simulink model "l8_ver130"  (Simulink 7.9)
settings: Solver=FixedStepDiscrete  SolverMode=SingleTasking  StartTime=0.0  StopTime=10  RelTol=1e-3
undefined symbols (not defined in the file or in its folder): sfp
libraries: xbsIndex_r4 x20466, casper_library_misc x43, dspsigops x32, simulink x7, xps_library x5, casper_library_flow_control x4
(part 50 of 210)

== system l8_ver130/s2/Halfband3/adder_tree ==
  en_in : Inport
  din1 : Inport  Port=2
  din2 : Inport  Port=3
  din3 : Inport  Port=4
  din4 : Inport  Port=5
  din5 : Inport  Port=6
  din6 : Inport  Port=7
  din7 : Inport  Port=8
  din8 : Inport  Port=9
  din9 : Inport  Port=10
  addr1 : Subsystem
      settings: AttributesFormatString=|
      | hold period: 2
      |  add latency: 1
  addr11 : Subsystem
      settings: AttributesFormatString=|
      | hold period: 2
      |  add latency: 1
  addr2 : Subsystem
      settings: AttributesFormatString=|
      | hold period: 2
      |  add latency: 1
  addr3 : Subsystem
      settings: AttributesFormatString=|
      | hold period: 2
      |  add latency: 1
  addr4 : Subsystem
      settings: AttributesFormatString=|
      | hold period: 2
      |  add latency: 1
  addr6 : Subsystem
      settings: AttributesFormatString=|
      | hold period: 2
      |  add latency: 1
  addr7 : Subsystem
      settings: AttributesFormatString=|
      | hold period: 2
      |  add latency: 1
  addr9 : Subsystem
      settings: AttributesFormatString=|
      | hold period: 2
      |  add latency: 1
  dly10 : xbsIndex_r4/Delay  rst=off  infoeditControl=Selection of Reset will increase slice count due to use of real FFs and instead of SRLs  en=off  latency=2  dbl_ovrd=off  reg_retiming=on  block_type=delay
  dly5 : xbsIndex_r4/Delay  rst=off  infoeditControl=Selection of Reset will increase slice count due to use of real FFs and instead of SRLs  en=off  latency=2  dbl_ovrd=off  reg_retiming=on  block_type=delay
  dly8 : xbsIndex_r4/Delay  rst=off  infoeditControl=Selection of Reset will increase slice count due to use of real FFs and instead of SRLs  en=off  latency=2  dbl_ovrd=off  reg_retiming=on  block_type=delay
  sync_delay : xbsIndex_r4/Delay  rst=off  infoeditControl=Selection of Reset will increase slice count due to use of real FFs and instead of SRLs  en=off  latency=4  dbl_ovrd=off  reg_retiming=on  block_type=delay
  en_out : Outport
  dout : Outport  Port=2
  wires:
  en_in/1 -> addr11/3(en_in), addr9/3(en_in), addr7/3(en_in), addr6/3(en_in), addr4/3(en_in), addr3/3(en_in), addr2/3(en_in), addr1/3(en_in), sync_delay/1
  addr11/1(sum_ab) -> dout/1  "adder_outs4_1"
  dly10/1 -> addr11/2(data_b)  "adder_outs3_2"
  addr9/1(sum_ab) -> addr11/1(data_a)  "adder_outs3_1"
  dly8/1 -> dly10/1  "adder_outs2_3"
  addr7/1(sum_ab) -> addr9/2(data_b)  "adder_outs2_2"
  addr6/1(sum_ab) -> addr9/1(data_a)  "adder_outs2_1"
  dly5/1 -> dly8/1  "adder_outs1_5"
  addr4/1(sum_ab) -> addr7/2(data_b)  "adder_outs1_4"
  addr3/1(sum_ab) -> addr7/1(data_a)  "adder_outs1_3"
  addr2/1(sum_ab) -> addr6/2(data_b)  "adder_outs1_2"
  addr1/1(sum_ab) -> addr6/1(data_a)  "adder_outs1_1"
  din9/1 -> dly5/1
  din8/1 -> addr4/2(data_b)
  din7/1 -> addr4/1(data_a)
  din6/1 -> addr3/2(data_b)
  din5/1 -> addr3/1(data_a)
  din4/1 -> addr2/2(data_b)
  din3/1 -> addr2/1(data_a)
  din2/1 -> addr1/2(data_b)
  din1/1 -> addr1/1(data_a)
  sync_delay/1 -> en_out/1

== system l8_ver130/s2/Halfband3/en_gen ==
  sync_in : Inport
  Constant : xbsIndex_r4/Constant  const=0  gui_display_data_type=Fixed-point  arith_type=Signed (2's comp)  n_bits=16  bin_pt=0  preci_type=Single  exp_width=8  frac_width=24  explicit_period=on  period=1  equ=P=C  opselect=C  inp2=PCIN>>17  opr=+  inp1=P  carry=CIN  dbl_ovrd=off  block_type=constant
  Counter1 : xbsIndex_r4/Counter  cnt_type=Count Limited  cnt_to=0  operation=Down  start_count=1  cnt_by_val=1  arith_type=Unsigned  n_bits=1  bin_pt=0  load_pin=off  rst=on  en=off  explicit_period=on  period=1  dbl_ovrd=off  use_behavioral_HDL=off  implementation=Fabric  block_type=counter
  Counter_delay : xbsIndex_r4/Delay  rst=off  infoeditControl=Selection of Reset will increase slice count due to use of real FFs and instead of SRLs  en=off  latency=0  dbl_ovrd=off  reg_retiming=off  block_type=delay
  Logical : xbsIndex_r4/Logical  logical_function=OR  inputs=2  en=off  latency=1  precision=Full  arith_type=Unsigned  n_bits=16  bin_pt=0  align_bp=on  dbl_ovrd=off  block_type=logical
  Relational : xbsIndex_r4/Relational  mode=a=b  en=off  latency=1  dbl_ovrd=off  block_type=relational
  en_out : Outport
  wires:
  Logical/1 -> en_out/1, Counter1/1  "xlsub1_Logical_out1"
  Relational/1 -> Logical/1  "xlsub1_Relational_out1"
  Constant/1 -> Relational/2  "xlsub1_Constant_out1"
  Counter_delay/1 -> Relational/1  "counter_delay_out"
  sync_in/1 -> Logical/2
  Counter1/1 -> Counter_delay/1  "xlsub1_Counter1_out1"

== system l8_ver130/s2/Halfband3/hold_fir_tap1 ==
  data_in : Inport
  en_in : Inport  Port=2
  Mult : xbsIndex_r4/Mult  precision=Full  arith_type="Signed  (2's comp)"  n_bits=16  bin_pt=14  quantization=Truncate  overflow=Wrap  en=on  latency=3  dbl_ovrd=off  use_behavioral_HDL=off  opt=Speed  use_embedded=on  optimum_pipeline=off  pipeline=on  use_rpm=on  placement_style=Triangular  block_type=mult
  coefficient : xbsIndex_r4/Constant  const=-0.026472867764434  gui_display_data_type=Fixed-point  arith_type=Signed (2's comp)  n_bits=16  bin_pt=14  preci_type=Single  exp_width=8  frac_width=24  explicit_period=on  period=1  equ=P=C  opselect=C  inp2=PCIN>>17  opr=+  inp1=P  carry=CIN  dbl_ovrd=off  block_type=constant
  mult_out : Outport
  en_out : Outport  Port=2
  wires:
  en_in/1 -> en_out/1, Mult/3
  coefficient/1 -> Mult/1  "xlsub2_coefficient_out1"
  Mult/1 -> mult_out/1
  data_in/1 -> Mult/2

== system l8_ver130/s2/Halfband3/hold_fir_tap11 ==
  data_in : Inport
  en_in : Inport  Port=2
  Mult : xbsIndex_r4/Mult  precision=Full  arith_type="Signed  (2's comp)"  n_bits=16  bin_pt=14  quantization=Truncate  overflow=Wrap  en=on  latency=3  dbl_ovrd=off  use_behavioral_HDL=off  opt=Speed  use_embedded=on  optimum_pipeline=off  pipeline=on  use_rpm=on  placement_style=Triangular  block_type=mult
  coefficient : xbsIndex_r4/Constant  const=-0.0934071809736732  gui_display_data_type=Fixed-point  arith_type=Signed (2's comp)  n_bits=16  bin_pt=14  preci_type=Single  exp_width=8  frac_width=24  explicit_period=on  period=1  equ=P=C  opselect=C  inp2=PCIN>>17  opr=+  inp1=P  carry=CIN  dbl_ovrd=off  block_type=constant
  mult_out : Outport
  en_out : Outport  Port=2
  wires:
  en_in/1 -> en_out/1, Mult/3
  coefficient/1 -> Mult/1  "xlsub2_coefficient_out1"
  Mult/1 -> mult_out/1
  data_in/1 -> Mult/2

== system l8_ver130/s2/Halfband3/hold_fir_tap13 ==
  data_in : Inport
  en_in : Inport  Port=2
  Mult : xbsIndex_r4/Mult  precision=Full  arith_type="Signed  (2's comp)"  n_bits=16  bin_pt=14  quantization=Truncate  overflow=Wrap  en=on  latency=3  dbl_ovrd=off  use_behavioral_HDL=off  opt=Speed  use_embedded=on  optimum_pipeline=off  pipeline=on  use_rpm=on  placement_style=Triangular  block_type=mult
  coefficient : xbsIndex_r4/Constant  const=0.0440903441068704  gui_display_data_type=Fixed-point  arith_type=Signed (2's comp)  n_bits=16  bin_pt=14  preci_type=Single  exp_width=8  frac_width=24  explicit_period=on  period=1  equ=P=C  opselect=C  inp2=PCIN>>17  opr=+  inp1=P  carry=CIN  dbl_ovrd=off  block_type=constant
  mult_out : Outport
  en_out : Outport  Port=2
  wires:
  en_in/1 -> en_out/1, Mult/3
  coefficient/1 -> Mult/1  "xlsub2_coefficient_out1"
  Mult/1 -> mult_out/1
  data_in/1 -> Mult/2

== system l8_ver130/s2/Halfband3/hold_fir_tap15 ==
  data_in : Inport
  en_in : Inport  Port=2
  Mult : xbsIndex_r4/Mult  precision=Full  arith_type="Signed  (2's comp)"  n_bits=16  bin_pt=14  quantization=Truncate  overflow=Wrap  en=on  latency=3  dbl_ovrd=off  use_behavioral_HDL=off  opt=Speed  use_embedded=on  optimum_pipeline=off  pipeline=on  use_rpm=on  placement_style=Triangular  block_type=mult
  coefficient : xbsIndex_r4/Constant  const=-0.026472867764434  gui_display_data_type=Fixed-point  arith_type=Signed (2's comp)  n_bits=16  bin_pt=14  preci_type=Single  exp_width=8  frac_width=24  explicit_period=on  period=1  equ=P=C  opselect=C  inp2=PCIN>>17  opr=+  inp1=P  carry=CIN  dbl_ovrd=off  block_type=constant
  mult_out : Outport
  en_out : Outport  Port=2
  wires:
  en_in/1 -> en_out/1, Mult/3
  coefficient/1 -> Mult/1  "xlsub2_coefficient_out1"
  Mult/1 -> mult_out/1
  data_in/1 -> Mult/2

== system l8_ver130/s2/Halfband3/hold_fir_tap3 ==
  data_in : Inport
  en_in : Inport  Port=2
  Mult : xbsIndex_r4/Mult  precision=Full  arith_type="Signed  (2's comp)"  n_bits=16  bin_pt=14  quantization=Truncate  overflow=Wrap  en=on  latency=3  dbl_ovrd=off  use_behavioral_HDL=off  opt=Speed  use_embedded=on  optimum_pipeline=off  pipeline=on  use_rpm=on  placement_style=Triangular  block_type=mult
  coefficient : xbsIndex_r4/Constant  const=0.0440903441068704  gui_display_data_type=Fixed-point  arith_type=Signed (2's comp)  n_bits=16  bin_pt=14  preci_type=Single  exp_width=8  frac_width=24  explicit_period=on  period=1  equ=P=C  opselect=C  inp2=PCIN>>17  opr=+  inp1=P  carry=CIN  dbl_ovrd=off  block_type=constant
  mult_out : Outport
  en_out : Outport  Port=2
  wires:
  en_in/1 -> en_out/1, Mult/3
  coefficient/1 -> Mult/1  "xlsub2_coefficient_out1"
  Mult/1 -> mult_out/1
  data_in/1 -> Mult/2

== system l8_ver130/s2/Halfband3/hold_fir_tap5 ==
  data_in : Inport
  en_in : Inport  Port=2
  Mult : xbsIndex_r4/Mult  precision=Full  arith_type="Signed  (2's comp)"  n_bits=16  bin_pt=14  quantization=Truncate  overflow=Wrap  en=on  latency=3  dbl_ovrd=off  use_behavioral_HDL=off  opt=Speed  use_embedded=on  optimum_pipeline=off  pipeline=on  use_rpm=on  placement_style=Triangular  block_type=mult
  coefficient : xbsIndex_r4/Constant  const=-0.0934071809736732  gui_display_data_type=Fixed-point  arith_type=Signed (2's comp)  n_bits=16  bin_pt=14  preci_type=Single  exp_width=8  frac_width=24  explicit_period=on  period=1  equ=P=C  opselect=C  inp2=PCIN>>17  opr=+  inp1=P  carry=CIN  dbl_ovrd=off  block_type=constant
  mult_out : Outport
  en_out : Outport  Port=2
  wires:
  en_in/1 -> en_out/1, Mult/3
  coefficient/1 -> Mult/1  "xlsub2_coefficient_out1"
  Mult/1 -> mult_out/1
  data_in/1 -> Mult/2

== system l8_ver130/s2/Halfband3/hold_fir_tap7 ==
  data_in : Inport
  en_in : Inport  Port=2
  Mult : xbsIndex_r4/Mult  precision=Full  arith_type="Signed  (2's comp)"  n_bits=16  bin_pt=14  quantization=Truncate  overflow=Wrap  en=on  latency=3  dbl_ovrd=off  use_behavioral_HDL=off  opt=Speed  use_embedded=on  optimum_pipeline=off  pipeline=on  use_rpm=on  placement_style=Triangular  block_type=mult
  coefficient : xbsIndex_r4/Constant  const=0.313910410160698  gui_display_data_type=Fixed-point  arith_type=Signed (2's comp)  n_bits=16  bin_pt=14  preci_type=Single  exp_width=8  frac_width=24  explicit_period=on  period=1  equ=P=C  opselect=C  inp2=PCIN>>17  opr=+  inp1=P  carry=CIN  dbl_ovrd=off  block_type=constant
  mult_out : Outport
  en_out : Outport  Port=2
  wires:
  en_in/1 -> en_out/1, Mult/3
  coefficient/1 -> Mult/1  "xlsub2_coefficient_out1"
  Mult/1 -> mult_out/1
  data_in/1 -> Mult/2

== system l8_ver130/s2/Halfband3/hold_fir_tap8 ==
  data_in : Inport
  en_in : Inport  Port=2
  Mult : xbsIndex_r4/Mult  precision=Full  arith_type="Signed  (2's comp)"  n_bits=16  bin_pt=14  quantization=Truncate  overflow=Wrap  en=on  latency=3  dbl_ovrd=off  use_behavioral_HDL=off  opt=Speed  use_embedded=on  optimum_pipeline=off  pipeline=on  use_rpm=on  placement_style=Triangular  block_type=mult
  coefficient : xbsIndex_r4/Constant  const=0.5  gui_display_data_type=Fixed-point  arith_type=Signed (2's comp)  n_bits=16  bin_pt=14  preci_type=Single  exp_width=8  frac_width=24  explicit_period=on  period=1  equ=P=C  opselect=C  inp2=PCIN>>17  opr=+  inp1=P  carry=CIN  dbl_ovrd=off  block_type=constant
  mult_out : Outport
  en_out : Outport  Port=2
  wires:
  en_in/1 -> en_out/1, Mult/3
  coefficient/1 -> Mult/1  "xlsub2_coefficient_out1"
  Mult/1 -> mult_out/1
  data_in/1 -> Mult/2

== system l8_ver130/s2/Halfband3/hold_fir_tap9 ==
  data_in : Inport
  en_in : Inport  Port=2
  Mult : xbsIndex_r4/Mult  precision=Full  arith_type="Signed  (2's comp)"  n_bits=16  bin_pt=14  quantization=Truncate  overflow=Wrap  en=on  latency=3  dbl_ovrd=off  use_behavioral_HDL=off  opt=Speed  use_embedded=on  optimum_pipeline=off  pipeline=on  use_rpm=on  placement_style=Triangular  block_type=mult
  coefficient : xbsIndex_r4/Constant  const=0.313910410160698  gui_display_data_type=Fixed-point  arith_type=Signed (2's comp)  n_bits=16  bin_pt=14  preci_type=Single  exp_width=8  frac_width=24  explicit_period=on  period=1  equ=P=C  opselect=C  inp2=PCIN>>17  opr=+  inp1=P  carry=CIN  dbl_ovrd=off  block_type=constant
  mult_out : Outport
  en_out : Outport  Port=2
  wires:
  en_in/1 -> en_out/1, Mult/3
  coefficient/1 -> Mult/1  "xlsub2_coefficient_out1"
  Mult/1 -> mult_out/1
  data_in/1 -> Mult/2

== system l8_ver130/s2/cic_pow2_0/Stage1_dec2 ==
  in1 : Inport
  in2 : Inport  Port=2
  in3 : Inport  Port=3
  in4 : Inport  Port=4
  in5 : Inport  Port=5
  in6 : Inport  Port=6
  in7 : Inport  Port=7
  in8 : Inport  Port=8
  in9 : Inport  Port=9
  in10 : Inport  Port=10
  in11 : Inport  Port=11
  in12 : Inport  Port=12
  in13 : Inport  Port=13
  in14 : Inport  Port=14
  in15 : Inport  Port=15
  in16 : Inport  Port=16
  sync_in : Inport  Port=17
  p_adder1 : Subsystem
      settings: AttributesFormatString=|
      | Accumulation Length = 2
      | Add Latency = 1
      | Delay Length =1
  p_adder2 : Subsystem
      settings: AttributesFormatString=|
      | Accumulation Length = 2
      | Add Latency = 1
      | Delay Length =1
  p_adder3 : Subsystem
      settings: AttributesFormatString=|
      | Accumulation Length = 2
      | Add Latency = 1
      | Delay Length =1
  p_adder4 : Subsystem
      settings: AttributesFormatString=|
      | Accumulation Length = 2
      | Add Latency = 1
      | Delay Length =1
  out1 : Outport
  out2 : Outport  Port=2
  out3 : Outport  Port=3
  out4 : Outport  Port=4
  out5 : Outport  Port=5
  out6 : Outport  Port=6
  out7 : Outport  Port=7
  out8 : Outport  Port=8
  sync_out : Outport  Port=9
  wires:
  sync_in/1 -> p_adder4/1(sync_in), p_adder3/1(sync_in), p_adder2/1(sync_in), p_adder1/1(sync_in)
  p_adder4/1(sync_out) -> sync_out/1  "sync_in5"
  p_adder4/17(out16) -> out8/1  "pa_i5_16"
  p_adder4/15(out14) -> out7/1  "pa_i5_14"
  p_adder4/13(out12) -> out6/1  "pa_i5_12"
  p_adder4/11(out10) -> out5/1  "pa_i5_10"
  p_adder4/9(out8) -> out4/1  "pa_i5_8"
  p_adder4/7(out6) -> out3/1  "pa_i5_6"
  p_adder4/5(out4) -> out2/1  "pa_i5_4"
  p_adder4/3(out2) -> out1/1  "pa_i5_2"
  p_adder3/17(out16) -> p_adder4/17(in16)  "pa_i4_16"
  p_adder3/16(out15) -> p_adder4/16(in15)  "pa_i4_15"
  p_adder3/15(out14) -> p_adder4/15(in14)  "pa_i4_14"
  p_adder3/14(out13) -> p_adder4/14(in13)  "pa_i4_13"
  p_adder3/13(out12) -> p_adder4/13(in12)  "pa_i4_12"
  p_adder3/12(out11) -> p_adder4/12(in11)  "pa_i4_11"
  p_adder3/11(out10) -> p_adder4/11(in10)  "pa_i4_10"
  p_adder3/10(out9) -> p_adder4/10(in9)  "pa_i4_9"
  p_adder3/9(out8) -> p_adder4/9(in8)  "pa_i4_8"
  p_adder3/8(out7) -> p_adder4/8(in7)  "pa_i4_7"
  p_adder3/7(out6) -> p_adder4/7(in6)  "pa_i4_6"
  p_adder3/6(out5) -> p_adder4/6(in5)  "pa_i4_5"
  p_adder3/5(out4) -> p_adder4/5(in4)  "pa_i4_4"
  p_adder3/4(out3) -> p_adder4/4(in3)  "pa_i4_3"
  p_adder3/3(out2) -> p_adder4/3(in2)  "pa_i4_2"
  p_adder3/2(out1) -> p_adder4/2(in1)  "pa_i4_1"
  p_adder2/17(out16) -> p_adder3/17(in16)  "pa_i3_16"
  p_adder2/16(out15) -> p_adder3/16(in15)  "pa_i3_15"
  p_adder2/15(out14) -> p_adder3/15(in14)  "pa_i3_14"
  p_adder2/14(out13) -> p_adder3/14(in13)  "pa_i3_13"
  p_adder2/13(out12) -> p_adder3/13(in12)  "pa_i3_12"
  p_adder2/12(out11) -> p_adder3/12(in11)  "pa_i3_11"
  p_adder2/11(out10) -> p_adder3/11(in10)  "pa_i3_10"
  p_adder2/10(out9) -> p_adder3/10(in9)  "pa_i3_9"
  p_adder2/9(out8) -> p_adder3/9(in8)  "pa_i3_8"
  p_adder2/8(out7) -> p_adder3/8(in7)  "pa_i3_7"
  p_adder2/7(out6) -> p_adder3/7(in6)  "pa_i3_6"
  p_adder2/6(out5) -> p_adder3/6(in5)  "pa_i3_5"
  p_adder2/5(out4) -> p_adder3/5(in4)  "pa_i3_4"
  p_adder2/4(out3) -> p_adder3/4(in3)  "pa_i3_3"
  p_adder2/3(out2) -> p_adder3/3(in2)  "pa_i3_2"
  p_adder2/2(out1) -> p_adder3/2(in1)  "pa_i3_1"
  p_adder1/17(out16) -> p_adder2/17(in16)  "pa_i2_16"
  p_adder1/16(out15) -> p_adder2/16(in15)  "pa_i2_15"
  p_adder1/15(out14) -> p_adder2/15(in14)  "pa_i2_14"
  p_adder1/14(out13) -> p_adder2/14(in13)  "pa_i2_13"
  p_adder1/13(out12) -> p_adder2/13(in12)  "pa_i2_12"
  p_adder1/12(out11) -> p_adder2/12(in11)  "pa_i2_11"
  p_adder1/11(out10) -> p_adder2/11(in10)  "pa_i2_10"
  p_adder1/10(out9) -> p_adder2/10(in9)  "pa_i2_9"
  p_adder1/9(out8) -> p_adder2/9(in8)  "pa_i2_8"
  p_adder1/8(out7) -> p_adder2/8(in7)  "pa_i2_7"
  p_adder1/7(out6) -> p_adder2/7(in6)  "pa_i2_6"
  p_adder1/6(out5) -> p_adder2/6(in5)  "pa_i2_5"
  p_adder1/5(out4) -> p_adder2/5(in4)  "pa_i2_4"
  p_adder1/4(out3) -> p_adder2/4(in3)  "pa_i2_3"
  p_adder1/3(out2) -> p_adder2/3(in2)  "pa_i2_2"
  p_adder1/2(out1) -> p_adder2/2(in1)  "pa_i2_1"
  in16/1 -> p_adder1/17(in16)
  in15/1 -> p_adder1/16(in15)
  in14/1 -> p_adder1/15(in14)
  in13/1 -> p_adder1/14(in13)
  in12/1 -> p_adder1/13(in12)
  in11/1 -> p_adder1/12(in11)
  in10/1 -> p_adder1/11(in10)
  in9/1 -> p_adder1/10(in9)
  in8/1 -> p_adder1/9(in8)
  in7/1 -> p_adder1/8(in7)
  in6/1 -> p_adder1/7(in6)
  in5/1 -> p_adder1/6(in5)
  in4/1 -> p_adder1/5(in4)
  in3/1 -> p_adder1/4(in3)
  in2/1 -> p_adder1/3(in2)
  in1/1 -> p_adder1/2(in1)

== system l8_ver130/s2/cic_pow2_0/Stage2_dec2 ==
  in1 : Inport
  in2 : Inport  Port=2
  in3 : Inport  Port=3
  in4 : Inport  Port=4
  in5 : Inport  Port=5
  in6 : Inport  Port=6
  in7 : Inport  Port=7
  in8 : Inport  Port=8
  sync_in : Inport  Port=9
  p_adder1 : Subsystem
      settings: AttributesFormatString=|
      | Accumulation Length = 2
      | Add Latency = 1
      | Delay Length =1
  p_adder2 : Subsystem
      settings: AttributesFormatString=|
      | Accumulation Length = 2
      | Add Latency = 1
      | Delay Length =1
  p_adder3 : Subsystem
      settings: AttributesFormatString=|
      | Accumulation Length = 2
      | Add Latency = 1
      | Delay Length =1
  p_adder4 : Subsystem
      settings: AttributesFormatString=|
      | Accumulation Length = 2
      | Add Latency = 1
      | Delay Length =1
  out1 : Outport
  out2 : Outport  Port=2
  out3 : Outport  Port=3
  out4 : Outport  Port=4
  sync_out : Outport  Port=5
  wires:
  sync_in/1 -> p_adder4/1(sync_in), p_adder3/1(sync_in), p_adder2/1(sync_in), p_adder1/1(sync_in)
  p_adder4/1(sync_out) -> sync_out/1  "sync_in5"
  p_adder4/9(out8) -> out4/1  "pa_i5_8"
  p_adder4/7(out6) -> out3/1  "pa_i5_6"
  p_adder4/5(out4) -> out2/1  "pa_i5_4"
  p_adder4/3(out2) -> out1/1  "pa_i5_2"
  p_adder3/9(out8) -> p_adder4/9(in8)  "pa_i4_8"
  p_adder3/8(out7) -> p_adder4/8(in7)  "pa_i4_7"
  p_adder3/7(out6) -> p_adder4/7(in6)  "pa_i4_6"
  p_adder3/6(out5) -> p_adder4/6(in5)  "pa_i4_5"
  p_adder3/5(out4) -> p_adder4/5(in4)  "pa_i4_4"
  p_adder3/4(out3) -> p_adder4/4(in3)  "pa_i4_3"
  p_adder3/3(out2) -> p_adder4/3(in2)  "pa_i4_2"
  p_adder3/2(out1) -> p_adder4/2(in1)  "pa_i4_1"
  p_adder2/9(out8) -> p_adder3/9(in8)  "pa_i3_8"
  p_adder2/8(out7) -> p_adder3/8(in7)  "pa_i3_7"
  p_adder2/7(out6) -> p_adder3/7(in6)  "pa_i3_6"
  p_adder2/6(out5) -> p_adder3/6(in5)  "pa_i3_5"
  p_adder2/5(out4) -> p_adder3/5(in4)  "pa_i3_4"
  p_adder2/4(out3) -> p_adder3/4(in3)  "pa_i3_3"
  p_adder2/3(out2) -> p_adder3/3(in2)  "pa_i3_2"
  p_adder2/2(out1) -> p_adder3/2(in1)  "pa_i3_1"
  p_adder1/9(out8) -> p_adder2/9(in8)  "pa_i2_8"
  p_adder1/8(out7) -> p_adder2/8(in7)  "pa_i2_7"
  p_adder1/7(out6) -> p_adder2/7(in6)  "pa_i2_6"
  p_adder1/6(out5) -> p_adder2/6(in5)  "pa_i2_5"
  p_adder1/5(out4) -> p_adder2/5(in4)  "pa_i2_4"
  p_adder1/4(out3) -> p_adder2/4(in3)  "pa_i2_3"
  p_adder1/3(out2) -> p_adder2/3(in2)  "pa_i2_2"
  p_adder1/2(out1) -> p_adder2/2(in1)  "pa_i2_1"
  in8/1 -> p_adder1/9(in8)
  in7/1 -> p_adder1/8(in7)
  in6/1 -> p_adder1/7(in6)
  in5/1 -> p_adder1/6(in5)
  in4/1 -> p_adder1/5(in4)
  in3/1 -> p_adder1/4(in3)
  in2/1 -> p_adder1/3(in2)
  in1/1 -> p_adder1/2(in1)

== system l8_ver130/s2/cic_pow2_0/Stage3_dec2 ==
  in1 : Inport
  in2 : Inport  Port=2
  in3 : Inport  Port=3
  in4 : Inport  Port=4
  sync_in : Inport  Port=5
  p_adder1 : Subsystem
      settings: AttributesFormatString=|
      | Accumulation Length = 2
      | Add Latency = 1
      | Delay Length =1
  p_adder2 : Subsystem
      settings: AttributesFormatString=|
      | Accumulation Length = 2
      | Add Latency = 1
      | Delay Length =1
  p_adder3 : Subsystem
      settings: AttributesFormatString=|
      | Accumulation Length = 2
      | Add Latency = 1
      | Delay Length =1
  p_adder4 : Subsystem
      settings: AttributesFormatString=|
      | Accumulation Length = 2
      | Add Latency = 1
      | Delay Length =1
  out1 : Outport
  out2 : Outport  Port=2
  sync_out : Outport  Port=3
  wires:
  sync_in/1 -> p_adder4/1(sync_in), p_adder3/1(sync_in), p_adder2/1(sync_in), p_adder1/1(sync_in)
  p_adder4/1(sync_out) -> sync_out/1  "sync_in5"
  p_adder4/5(out4) -> out2/1  "pa_i5_4"
  p_adder4/3(out2) -> out1/1  "pa_i5_2"
  p_adder3/5(out4) -> p_adder4/5(in4)  "pa_i4_4"
  p_adder3/4(out3) -> p_adder4/4(in3)  "pa_i4_3"
  p_adder3/3(out2) -> p_adder4/3(in2)  "pa_i4_2"
  p_adder3/2(out1) -> p_adder4/2(in1)  "pa_i4_1"
  p_adder2/5(out4) -> p_adder3/5(in4)  "pa_i3_4"
  p_adder2/4(out3) -> p_adder3/4(in3)  "pa_i3_3"
  p_adder2/3(out2) -> p_adder3/3(in2)  "pa_i3_2"
  p_adder2/2(out1) -> p_adder3/2(in1)  "pa_i3_1"
  p_adder1/5(out4) -> p_adder2/5(in4)  "pa_i2_4"
  p_adder1/4(out3) -> p_adder2/4(in3)  "pa_i2_3"
  p_adder1/3(out2) -> p_adder2/3(in2)  "pa_i2_2"
  p_adder1/2(out1) -> p_adder2/2(in1)  "pa_i2_1"
  in4/1 -> p_adder1/5(in4)
  in3/1 -> p_adder1/4(in3)
  in2/1 -> p_adder1/3(in2)
  in1/1 -> p_adder1/2(in1)

== system l8_ver130/s2/cic_pow2_0/Stage4_dec2 ==
  in1 : Inport
  in2 : Inport  Port=2
  sync_in : Inport  Port=3
  p_adder1 : Subsystem
      settings: AttributesFormatString=|
      | Accumulation Length = 2
      | Add Latency = 1
      | Delay Length =1
  p_adder2 : Subsystem
      settings: AttributesFormatString=|
      | Accumulation Length = 2
      | Add Latency = 1
      | Delay Length =1
  p_adder3 : Subsystem
      settings: AttributesFormatString=|
      | Accumulation Length = 2
      | Add Latency = 1
      | Delay Length =1
  p_adder4 : Subsystem
      settings: AttributesFormatString=|
      | Accumulation Length = 2
      | Add Latency = 1
      | Delay Length =1
  out1 : Outport
  sync_out : Outport  Port=2
  wires:
  sync_in/1 -> p_adder4/1(sync_in), p_adder3/1(sync_in), p_adder2/1(sync_in), p_adder1/1(sync_in)
  p_adder4/1(sync_out) -> sync_out/1  "sync_in5"
  p_adder4/3(out2) -> out1/1  "pa_i5_2"
  p_adder3/3(out2) -> p_adder4/3(in2)  "pa_i4_2"
  p_adder3/2(out1) -> p_adder4/2(in1)  "pa_i4_1"
  p_adder2/3(out2) -> p_adder3/3(in2)  "pa_i3_2"
  p_adder2/2(out1) -> p_adder3/2(in1)  "pa_i3_1"
  p_adder1/3(out2) -> p_adder2/3(in2)  "pa_i2_2"
  p_adder1/2(out1) -> p_adder2/2(in1)  "pa_i2_1"
  in2/1 -> p_adder1/3(in2)
  in1/1 -> p_adder1/2(in1)

== system l8_ver130/s2/cic_pow2_1/Stage1_dec2 ==
  in1 : Inport
  in2 : Inport  Port=2
  in3 : Inport  Port=3
  in4 : Inport  Port=4
  in5 : Inport  Port=5
  in6 : Inport  Port=6
  in7 : Inport  Port=7
  in8 : Inport  Port=8
  in9 : Inport  Port=9
  in10 : Inport  Port=10
  in11 : Inport  Port=11
  in12 : Inport  Port=12
  in13 : Inport  Port=13
  in14 : Inport  Port=14
  in15 : Inport  Port=15
  in16 : Inport  Port=16
  sync_in : Inport  Port=17
  p_adder1 : Subsystem
      settings: AttributesFormatString=|
      | Accumulation Length = 2
      | Add Latency = 1
      | Delay Length =1
  p_adder2 : Subsystem
      settings: AttributesFormatString=|
      | Accumulation Length = 2
      | Add Latency = 1
      | Delay Length =1
  p_adder3 : Subsystem
      settings: AttributesFormatString=|
      | Accumulation Length = 2
      | Add Latency = 1
      | Delay Length =1
  p_adder4 : Subsystem
      settings: AttributesFormatString=|
      | Accumulation Length = 2
      | Add Latency = 1
      | Delay Length =1
  out1 : Outport
  out2 : Outport  Port=2
  out3 : Outport  Port=3
  out4 : Outport  Port=4
  out5 : Outport  Port=5
  out6 : Outport  Port=6
  out7 : Outport  Port=7
  out8 : Outport  Port=8
  sync_out : Outport  Port=9
  wires:
  sync_in/1 -> p_adder4/1(sync_in), p_adder3/1(sync_in), p_adder2/1(sync_in), p_adder1/1(sync_in)
  p_adder4/1(sync_out) -> sync_out/1  "sync_in5"
  p_adder4/17(out16) -> out8/1  "pa_i5_16"
  p_adder4/15(out14) -> out7/1  "pa_i5_14"
  p_adder4/13(out12) -> out6/1  "pa_i5_12"
  p_adder4/11(out10) -> out5/1  "pa_i5_10"
  p_adder4/9(out8) -> out4/1  "pa_i5_8"
  p_adder4/7(out6) -> out3/1  "pa_i5_6"
  p_adder4/5(out4) -> out2/1  "pa_i5_4"
  p_adder4/3(out2) -> out1/1  "pa_i5_2"
  p_adder3/17(out16) -> p_adder4/17(in16)  "pa_i4_16"
  p_adder3/16(out15) -> p_adder4/16(in15)  "pa_i4_15"
  p_adder3/15(out14) -> p_adder4/15(in14)  "pa_i4_14"
  p_adder3/14(out13) -> p_adder4/14(in13)  "pa_i4_13"
  p_adder3/13(out12) -> p_adder4/13(in12)  "pa_i4_12"
  p_adder3/12(out11) -> p_adder4/12(in11)  "pa_i4_11"
  p_adder3/11(out10) -> p_adder4/11(in10)  "pa_i4_10"
  p_adder3/10(out9) -> p_adder4/10(in9)  "pa_i4_9"
  p_adder3/9(out8) -> p_adder4/9(in8)  "pa_i4_8"
  p_adder3/8(out7) -> p_adder4/8(in7)  "pa_i4_7"
  p_adder3/7(out6) -> p_adder4/7(in6)  "pa_i4_6"
  p_adder3/6(out5) -> p_adder4/6(in5)  "pa_i4_5"
  p_adder3/5(out4) -> p_adder4/5(in4)  "pa_i4_4"
  p_adder3/4(out3) -> p_adder4/4(in3)  "pa_i4_3"
  p_adder3/3(out2) -> p_adder4/3(in2)  "pa_i4_2"
  p_adder3/2(out1) -> p_adder4/2(in1)  "pa_i4_1"
  p_adder2/17(out16) -> p_adder3/17(in16)  "pa_i3_16"
  p_adder2/16(out15) -> p_adder3/16(in15)  "pa_i3_15"
  p_adder2/15(out14) -> p_adder3/15(in14)  "pa_i3_14"
  p_adder2/14(out13) -> p_adder3/14(in13)  "pa_i3_13"
  p_adder2/13(out12) -> p_adder3/13(in12)  "pa_i3_12"
  p_adder2/12(out11) -> p_adder3/12(in11)  "pa_i3_11"
  p_adder2/11(out10) -> p_adder3/11(in10)  "pa_i3_10"
  p_adder2/10(out9) -> p_adder3/10(in9)  "pa_i3_9"
  p_adder2/9(out8) -> p_adder3/9(in8)  "pa_i3_8"
  p_adder2/8(out7) -> p_adder3/8(in7)  "pa_i3_7"
  p_adder2/7(out6) -> p_adder3/7(in6)  "pa_i3_6"
  p_adder2/6(out5) -> p_adder3/6(in5)  "pa_i3_5"
  p_adder2/5(out4) -> p_adder3/5(in4)  "pa_i3_4"
  p_adder2/4(out3) -> p_adder3/4(in3)  "pa_i3_3"
  p_adder2/3(out2) -> p_adder3/3(in2)  "pa_i3_2"
  p_adder2/2(out1) -> p_adder3/2(in1)  "pa_i3_1"
  p_adder1/17(out16) -> p_adder2/17(in16)  "pa_i2_16"
  p_adder1/16(out15) -> p_adder2/16(in15)  "pa_i2_15"
  p_adder1/15(out14) -> p_adder2/15(in14)  "pa_i2_14"
  p_adder1/14(out13) -> p_adder2/14(in13)  "pa_i2_13"
  p_adder1/13(out12) -> p_adder2/13(in12)  "pa_i2_12"
  p_adder1/12(out11) -> p_adder2/12(in11)  "pa_i2_11"
  p_adder1/11(out10) -> p_adder2/11(in10)  "pa_i2_10"
  p_adder1/10(out9) -> p_adder2/10(in9)  "pa_i2_9"
  p_adder1/9(out8) -> p_adder2/9(in8)  "pa_i2_8"
  p_adder1/8(out7) -> p_adder2/8(in7)  "pa_i2_7"
  p_adder1/7(out6) -> p_adder2/7(in6)  "pa_i2_6"
  p_adder1/6(out5) -> p_adder2/6(in5)  "pa_i2_5"
  p_adder1/5(out4) -> p_adder2/5(in4)  "pa_i2_4"
  p_adder1/4(out3) -> p_adder2/4(in3)  "pa_i2_3"
  p_adder1/3(out2) -> p_adder2/3(in2)  "pa_i2_2"
  p_adder1/2(out1) -> p_adder2/2(in1)  "pa_i2_1"
  in16/1 -> p_adder1/17(in16)
  in15/1 -> p_adder1/16(in15)
  in14/1 -> p_adder1/15(in14)
  in13/1 -> p_adder1/14(in13)
  in12/1 -> p_adder1/13(in12)
  in11/1 -> p_adder1/12(in11)
  in10/1 -> p_adder1/11(in10)
  in9/1 -> p_adder1/10(in9)
  in8/1 -> p_adder1/9(in8)
  in7/1 -> p_adder1/8(in7)
  in6/1 -> p_adder1/7(in6)
  in5/1 -> p_adder1/6(in5)
  in4/1 -> p_adder1/5(in4)
  in3/1 -> p_adder1/4(in3)
  in2/1 -> p_adder1/3(in2)
  in1/1 -> p_adder1/2(in1)

== system l8_ver130/s2/cic_pow2_1/Stage2_dec2 ==
  in1 : Inport
  in2 : Inport  Port=2
  in3 : Inport  Port=3
  in4 : Inport  Port=4
  in5 : Inport  Port=5
  in6 : Inport  Port=6
  in7 : Inport  Port=7
  in8 : Inport  Port=8
  sync_in : Inport  Port=9
  p_adder1 : Subsystem
      settings: AttributesFormatString=|
      | Accumulation Length = 2
      | Add Latency = 1
      | Delay Length =1
  p_adder2 : Subsystem
      settings: AttributesFormatString=|
      | Accumulation Length = 2
      | Add Latency = 1
      | Delay Length =1
  p_adder3 : Subsystem
      settings: AttributesFormatString=|
      | Accumulation Length = 2
      | Add Latency = 1
      | Delay Length =1
  p_adder4 : Subsystem
      settings: AttributesFormatString=|
      | Accumulation Length = 2
      | Add Latency = 1
      | Delay Length =1
  out1 : Outport
  out2 : Outport  Port=2
  out3 : Outport  Port=3
  out4 : Outport  Port=4
  sync_out : Outport  Port=5
  wires:
  sync_in/1 -> p_adder4/1(sync_in), p_adder3/1(sync_in), p_adder2/1(sync_in), p_adder1/1(sync_in)
  p_adder4/1(sync_out) -> sync_out/1  "sync_in5"
  p_adder4/9(out8) -> out4/1  "pa_i5_8"
  p_adder4/7(out6) -> out3/1  "pa_i5_6"
  p_adder4/5(out4) -> out2/1  "pa_i5_4"
  p_adder4/3(out2) -> out1/1  "pa_i5_2"
  p_adder3/9(out8) -> p_adder4/9(in8)  "pa_i4_8"
  p_adder3/8(out7) -> p_adder4/8(in7)  "pa_i4_7"
  p_adder3/7(out6) -> p_adder4/7(in6)  "pa_i4_6"
  p_adder3/6(out5) -> p_adder4/6(in5)  "pa_i4_5"
  p_adder3/5(out4) -> p_adder4/5(in4)  "pa_i4_4"
  p_adder3/4(out3) -> p_adder4/4(in3)  "pa_i4_3"
  p_adder3/3(out2) -> p_adder4/3(in2)  "pa_i4_2"
  p_adder3/2(out1) -> p_adder4/2(in1)  "pa_i4_1"
  p_adder2/9(out8) -> p_adder3/9(in8)  "pa_i3_8"
  p_adder2/8(out7) -> p_adder3/8(in7)  "pa_i3_7"
  p_adder2/7(out6) -> p_adder3/7(in6)  "pa_i3_6"
  p_adder2/6(out5) -> p_adder3/6(in5)  "pa_i3_5"
  p_adder2/5(out4) -> p_adder3/5(in4)  "pa_i3_4"
  p_adder2/4(out3) -> p_adder3/4(in3)  "pa_i3_3"
  p_adder2/3(out2) -> p_adder3/3(in2)  "pa_i3_2"
  p_adder2/2(out1) -> p_adder3/2(in1)  "pa_i3_1"
  p_adder1/9(out8) -> p_adder2/9(in8)  "pa_i2_8"
  p_adder1/8(out7) -> p_adder2/8(in7)  "pa_i2_7"
  p_adder1/7(out6) -> p_adder2/7(in6)  "pa_i2_6"
  p_adder1/6(out5) -> p_adder2/6(in5)  "pa_i2_5"
  p_adder1/5(out4) -> p_adder2/5(in4)  "pa_i2_4"
  p_adder1/4(out3) -> p_adder2/4(in3)  "pa_i2_3"
  p_adder1/3(out2) -> p_adder2/3(in2)  "pa_i2_2"
  p_adder1/2(out1) -> p_adder2/2(in1)  "pa_i2_1"
  in8/1 -> p_adder1/9(in8)
  in7/1 -> p_adder1/8(in7)
  in6/1 -> p_adder1/7(in6)
  in5/1 -> p_adder1/6(in5)
  in4/1 -> p_adder1/5(in4)
  in3/1 -> p_adder1/4(in3)
  in2/1 -> p_adder1/3(in2)
  in1/1 -> p_adder1/2(in1)

== system l8_ver130/s2/cic_pow2_1/Stage3_dec2 ==
  in1 : Inport
  in2 : Inport  Port=2
  in3 : Inport  Port=3
  in4 : Inport  Port=4
  sync_in : Inport  Port=5
  p_adder1 : Subsystem
      settings: AttributesFormatString=|
      | Accumulation Length = 2
      | Add Latency = 1
      | Delay Length =1
  p_adder2 : Subsystem
      settings: AttributesFormatString=|
      | Accumulation Length = 2
      | Add Latency = 1
      | Delay Length =1
  p_adder3 : Subsystem
      settings: AttributesFormatString=|
      | Accumulation Length = 2
      | Add Latency = 1
      | Delay Length =1
  p_adder4 : Subsystem
      settings: AttributesFormatString=|
      | Accumulation Length = 2
      | Add Latency = 1
      | Delay Length =1
  out1 : Outport
  out2 : Outport  Port=2
  sync_out : Outport  Port=3
  wires:
  sync_in/1 -> p_adder4/1(sync_in), p_adder3/1(sync_in), p_adder2/1(sync_in), p_adder1/1(sync_in)
  p_adder4/1(sync_out) -> sync_out/1  "sync_in5"
  p_adder4/5(out4) -> out2/1  "pa_i5_4"
  p_adder4/3(out2) -> out1/1  "pa_i5_2"
  p_adder3/5(out4) -> p_adder4/5(in4)  "pa_i4_4"
  p_adder3/4(out3) -> p_adder4/4(in3)  "pa_i4_3"
  p_adder3/3(out2) -> p_adder4/3(in2)  "pa_i4_2"
  p_adder3/2(out1) -> p_adder4/2(in1)  "pa_i4_1"
  p_adder2/5(out4) -> p_adder3/5(in4)  "pa_i3_4"
  p_adder2/4(out3) -> p_adder3/4(in3)  "pa_i3_3"
  p_adder2/3(out2) -> p_adder3/3(in2)  "pa_i3_2"
  p_adder2/2(out1) -> p_adder3/2(in1)  "pa_i3_1"
  p_adder1/5(out4) -> p_adder2/5(in4)  "pa_i2_4"
  p_adder1/4(out3) -> p_adder2/4(in3)  "pa_i2_3"
  p_adder1/3(out2) -> p_adder2/3(in2)  "pa_i2_2"
  p_adder1/2(out1) -> p_adder2/2(in1)  "pa_i2_1"
  in4/1 -> p_adder1/5(in4)
  in3/1 -> p_adder1/4(in3)
  in2/1 -> p_adder1/3(in2)
  in1/1 -> p_adder1/2(in1)

== system l8_ver130/s2/cic_pow2_1/Stage4_dec2 ==
  in1 : Inport
  in2 : Inport  Port=2
  sync_in : Inport  Port=3
  p_adder1 : Subsystem
      settings: AttributesFormatString=|
      | Accumulation Length = 2
      | Add Latency = 1
      | Delay Length =1
  p_adder2 : Subsystem
      settings: AttributesFormatString=|
      | Accumulation Length = 2
      | Add Latency = 1
      | Delay Length =1
  p_adder3 : Subsystem
      settings: AttributesFormatString=|
      | Accumulation Length = 2
      | Add Latency = 1
      | Delay Length =1
  p_adder4 : Subsystem
      settings: AttributesFormatString=|
      | Accumulation Length = 2
      | Add Latency = 1
      | Delay Length =1
  out1 : Outport
  sync_out : Outport  Port=2
  wires:
  sync_in/1 -> p_adder4/1(sync_in), p_adder3/1(sync_in), p_adder2/1(sync_in), p_adder1/1(sync_in)
  p_adder4/1(sync_out) -> sync_out/1  "sync_in5"
  p_adder4/3(out2) -> out1/1  "pa_i5_2"
  p_adder3/3(out2) -> p_adder4/3(in2)  "pa_i4_2"
  p_adder3/2(out1) -> p_adder4/2(in1)  "pa_i4_1"
  p_adder2/3(out2) -> p_adder3/3(in2)  "pa_i3_2"
  p_adder2/2(out1) -> p_adder3/2(in1)  "pa_i3_1"
  p_adder1/3(out2) -> p_adder2/3(in2)  "pa_i2_2"
  p_adder1/2(out1) -> p_adder2/2(in1)  "pa_i2_1"
  in2/1 -> p_adder1/3(in2)
  in1/1 -> p_adder1/2(in1)

== system l8_ver130/s2/cic_pow2_2/Stage1_dec2 ==
  in1 : Inport
  in2 : Inport  Port=2
  in3 : Inport  Port=3
  in4 : Inport  Port=4
  in5 : Inport  Port=5
  in6 : Inport  Port=6
  in7 : Inport  Port=7
  in8 : Inport  Port=8
  in9 : Inport  Port=9
  in10 : Inport  Port=10
  in11 : Inport  Port=11
  in12 : Inport  Port=12
  in13 : Inport  Port=13
  in14 : Inport  Port=14
  in15 : Inport  Port=15
  in16 : Inport  Port=16
  sync_in : Inport  Port=17
  p_adder1 : Subsystem
      settings: AttributesFormatString=|
      | Accumulation Length = 2
      | Add Latency = 1
      | Delay Length =1
  p_adder2 : Subsystem
      settings: AttributesFormatString=|
      | Accumulation Length = 2
      | Add Latency = 1
      | Delay Length =1
  p_adder3 : Subsystem
      settings: AttributesFormatString=|
      | Accumulation Length = 2
      | Add Latency = 1
      | Delay Length =1
  p_adder4 : Subsystem
      settings: AttributesFormatString=|
      | Accumulation Length = 2
      | Add Latency = 1
      | Delay Length =1
  out1 : Outport
  out2 : Outport  Port=2
  out3 : Outport  Port=3
  out4 : Outport  Port=4
  out5 : Outport  Port=5
  out6 : Outport  Port=6
  out7 : Outport  Port=7
  out8 : Outport  Port=8
  sync_out : Outport  Port=9
  wires:
  sync_in/1 -> p_adder4/1(sync_in), p_adder3/1(sync_in), p_adder2/1(sync_in), p_adder1/1(sync_in)
  p_adder4/1(sync_out) -> sync_out/1  "sync_in5"
  p_adder4/17(out16) -> out8/1  "pa_i5_16"
  p_adder4/15(out14) -> out7/1  "pa_i5_14"
  p_adder4/13(out12) -> out6/1  "pa_i5_12"
  p_adder4/11(out10) -> out5/1  "pa_i5_10"
  p_adder4/9(out8) -> out4/1  "pa_i5_8"
  p_adder4/7(out6) -> out3/1  "pa_i5_6"
  p_adder4/5(out4) -> out2/1  "pa_i5_4"
  p_adder4/3(out2) -> out1/1  "pa_i5_2"
  p_adder3/17(out16) -> p_adder4/17(in16)  "pa_i4_16"
  p_adder3/16(out15) -> p_adder4/16(in15)  "pa_i4_15"
  p_adder3/15(out14) -> p_adder4/15(in14)  "pa_i4_14"
  p_adder3/14(out13) -> p_adder4/14(in13)  "pa_i4_13"
  p_adder3/13(out12) -> p_adder4/13(in12)  "pa_i4_12"
  p_adder3/12(out11) -> p_adder4/12(in11)  "pa_i4_11"
  p_adder3/11(out10) -> p_adder4/11(in10)  "pa_i4_10"
  p_adder3/10(out9) -> p_adder4/10(in9)  "pa_i4_9"
  p_adder3/9(out8) -> p_adder4/9(in8)  "pa_i4_8"
  p_adder3/8(out7) -> p_adder4/8(in7)  "pa_i4_7"
  p_adder3/7(out6) -> p_adder4/7(in6)  "pa_i4_6"
  p_adder3/6(out5) -> p_adder4/6(in5)  "pa_i4_5"
  p_adder3/5(out4) -> p_adder4/5(in4)  "pa_i4_4"
  p_adder3/4(out3) -> p_adder4/4(in3)  "pa_i4_3"
  p_adder3/3(out2) -> p_adder4/3(in2)  "pa_i4_2"
  p_adder3/2(out1) -> p_adder4/2(in1)  "pa_i4_1"
  p_adder2/17(out16) -> p_adder3/17(in16)  "pa_i3_16"
  p_adder2/16(out15) -> p_adder3/16(in15)  "pa_i3_15"
  p_adder2/15(out14) -> p_adder3/15(in14)  "pa_i3_14"
  p_adder2/14(out13) -> p_adder3/14(in13)  "pa_i3_13"
  p_adder2/13(out12) -> p_adder3/13(in12)  "pa_i3_12"
  p_adder2/12(out11) -> p_adder3/12(in11)  "pa_i3_11"
  p_adder2/11(out10) -> p_adder3/11(in10)  "pa_i3_10"
  p_adder2/10(out9) -> p_adder3/10(in9)  "pa_i3_9"
  p_adder2/9(out8) -> p_adder3/9(in8)  "pa_i3_8"
  p_adder2/8(out7) -> p_adder3/8(in7)  "pa_i3_7"
  p_adder2/7(out6) -> p_adder3/7(in6)  "pa_i3_6"
  p_adder2/6(out5) -> p_adder3/6(in5)  "pa_i3_5"
  p_adder2/5(out4) -> p_adder3/5(in4)  "pa_i3_4"
  p_adder2/4(out3) -> p_adder3/4(in3)  "pa_i3_3"
  p_adder2/3(out2) -> p_adder3/3(in2)  "pa_i3_2"
  p_adder2/2(out1) -> p_adder3/2(in1)  "pa_i3_1"
  p_adder1/17(out16) -> p_adder2/17(in16)  "pa_i2_16"
  p_adder1/16(out15) -> p_adder2/16(in15)  "pa_i2_15"
  p_adder1/15(out14) -> p_adder2/15(in14)  "pa_i2_14"
  p_adder1/14(out13) -> p_adder2/14(in13)  "pa_i2_13"
  p_adder1/13(out12) -> p_adder2/13(in12)  "pa_i2_12"
  p_adder1/12(out11) -> p_adder2/12(in11)  "pa_i2_11"
  p_adder1/11(out10) -> p_adder2/11(in10)  "pa_i2_10"
  p_adder1/10(out9) -> p_adder2/10(in9)  "pa_i2_9"
  p_adder1/9(out8) -> p_adder2/9(in8)  "pa_i2_8"
  p_adder1/8(out7) -> p_adder2/8(in7)  "pa_i2_7"
  p_adder1/7(out6) -> p_adder2/7(in6)  "pa_i2_6"
  p_adder1/6(out5) -> p_adder2/6(in5)  "pa_i2_5"
  p_adder1/5(out4) -> p_adder2/5(in4)  "pa_i2_4"
  p_adder1/4(out3) -> p_adder2/4(in3)  "pa_i2_3"
  p_adder1/3(out2) -> p_adder2/3(in2)  "pa_i2_2"
  p_adder1/2(out1) -> p_adder2/2(in1)  "pa_i2_1"
  in16/1 -> p_adder1/17(in16)
  in15/1 -> p_adder1/16(in15)
  in14/1 -> p_adder1/15(in14)
  in13/1 -> p_adder1/14(in13)
  in12/1 -> p_adder1/13(in12)
  in11/1 -> p_adder1/12(in11)
  in10/1 -> p_adder1/11(in10)
  in9/1 -> p_adder1/10(in9)
  in8/1 -> p_adder1/9(in8)
  in7/1 -> p_adder1/8(in7)
  in6/1 -> p_adder1/7(in6)
  in5/1 -> p_adder1/6(in5)
  in4/1 -> p_adder1/5(in4)
  in3/1 -> p_adder1/4(in3)
  in2/1 -> p_adder1/3(in2)
  in1/1 -> p_adder1/2(in1)

== system l8_ver130/s2/cic_pow2_2/Stage2_dec2 ==
  in1 : Inport
  in2 : Inport  Port=2
  in3 : Inport  Port=3
  in4 : Inport  Port=4
  in5 : Inport  Port=5
  in6 : Inport  Port=6
  in7 : Inport  Port=7
  in8 : Inport  Port=8
  sync_in : Inport  Port=9
  p_adder1 : Subsystem
      settings: AttributesFormatString=|
      | Accumulation Length = 2
      | Add Latency = 1
      | Delay Length =1
  p_adder2 : Subsystem
      settings: AttributesFormatString=|
      | Accumulation Length = 2
      | Add Latency = 1
      | Delay Length =1
  p_adder3 : Subsystem
      settings: AttributesFormatString=|
      | Accumulation Length = 2
      | Add Latency = 1
      | Delay Length =1
  p_adder4 : Subsystem
      settings: AttributesFormatString=|
      | Accumulation Length = 2
      | Add Latency = 1
      | Delay Length =1
  out1 : Outport
  out2 : Outport  Port=2
  out3 : Outport  Port=3
  out4 : Outport  Port=4
  sync_out : Outport  Port=5
  wires:
  sync_in/1 -> p_adder4/1(sync_in), p_adder3/1(sync_in), p_adder2/1(sync_in), p_adder1/1(sync_in)
  p_adder4/1(sync_out) -> sync_out/1  "sync_in5"
  p_adder4/9(out8) -> out4/1  "pa_i5_8"
  p_adder4/7(out6) -> out3/1  "pa_i5_6"
  p_adder4/5(out4) -> out2/1  "pa_i5_4"
  p_adder4/3(out2) -> out1/1  "pa_i5_2"
  p_adder3/9(out8) -> p_adder4/9(in8)  "pa_i4_8"
  p_adder3/8(out7) -> p_adder4/8(in7)  "pa_i4_7"
  p_adder3/7(out6) -> p_adder4/7(in6)  "pa_i4_6"
  p_adder3/6(out5) -> p_adder4/6(in5)  "pa_i4_5"
  p_adder3/5(out4) -> p_adder4/5(in4)  "pa_i4_4"
  p_adder3/4(out3) -> p_adder4/4(in3)  "pa_i4_3"
  p_adder3/3(out2) -> p_adder4/3(in2)  "pa_i4_2"
  p_adder3/2(out1) -> p_adder4/2(in1)  "pa_i4_1"
  p_adder2/9(out8) -> p_adder3/9(in8)  "pa_i3_8"
  p_adder2/8(out7) -> p_adder3/8(in7)  "pa_i3_7"
  p_adder2/7(out6) -> p_adder3/7(in6)  "pa_i3_6"
  p_adder2/6(out5) -> p_adder3/6(in5)  "pa_i3_5"
  p_adder2/5(out4) -> p_adder3/5(in4)  "pa_i3_4"
  p_adder2/4(out3) -> p_adder3/4(in3)  "pa_i3_3"
  p_adder2/3(out2) -> p_adder3/3(in2)  "pa_i3_2"
  p_adder2/2(out1) -> p_adder3/2(in1)  "pa_i3_1"
  p_adder1/9(out8) -> p_adder2/9(in8)  "pa_i2_8"
  p_adder1/8(out7) -> p_adder2/8(in7)  "pa_i2_7"
  p_adder1/7(out6) -> p_adder2/7(in6)  "pa_i2_6"
  p_adder1/6(out5) -> p_adder2/6(in5)  "pa_i2_5"
  p_adder1/5(out4) -> p_adder2/5(in4)  "pa_i2_4"
  p_adder1/4(out3) -> p_adder2/4(in3)  "pa_i2_3"
  p_adder1/3(out2) -> p_adder2/3(in2)  "pa_i2_2"
  p_adder1/2(out1) -> p_adder2/2(in1)  "pa_i2_1"
  in8/1 -> p_adder1/9(in8)
  in7/1 -> p_adder1/8(in7)
  in6/1 -> p_adder1/7(in6)
  in5/1 -> p_adder1/6(in5)
  in4/1 -> p_adder1/5(in4)
  in3/1 -> p_adder1/4(in3)
  in2/1 -> p_adder1/3(in2)
  in1/1 -> p_adder1/2(in1)

== system l8_ver130/s2/cic_pow2_2/Stage3_dec2 ==
  in1 : Inport
  in2 : Inport  Port=2
  in3 : Inport  Port=3
  in4 : Inport  Port=4
  sync_in : Inport  Port=5
  p_adder1 : Subsystem
      settings: AttributesFormatString=|
      | Accumulation Length = 2
      | Add Latency = 1
      | Delay Length =1
  p_adder2 : Subsystem
      settings: AttributesFormatString=|
      | Accumulation Length = 2
      | Add Latency = 1
      | Delay Length =1
  p_adder3 : Subsystem
      settings: AttributesFormatString=|
      | Accumulation Length = 2
      | Add Latency = 1
      | Delay Length =1
  p_adder4 : Subsystem
      settings: AttributesFormatString=|
      | Accumulation Length = 2
      | Add Latency = 1
      | Delay Length =1
  out1 : Outport
  out2 : Outport  Port=2
  sync_out : Outport  Port=3
  wires:
  sync_in/1 -> p_adder4/1(sync_in), p_adder3/1(sync_in), p_adder2/1(sync_in), p_adder1/1(sync_in)
  p_adder4/1(sync_out) -> sync_out/1  "sync_in5"
  p_adder4/5(out4) -> out2/1  "pa_i5_4"
  p_adder4/3(out2) -> out1/1  "pa_i5_2"
  p_adder3/5(out4) -> p_adder4/5(in4)  "pa_i4_4"
  p_adder3/4(out3) -> p_adder4/4(in3)  "pa_i4_3"
  p_adder3/3(out2) -> p_adder4/3(in2)  "pa_i4_2"
  p_adder3/2(out1) -> p_adder4/2(in1)  "pa_i4_1"
  p_adder2/5(out4) -> p_adder3/5(in4)  "pa_i3_4"
  p_adder2/4(out3) -> p_adder3/4(in3)  "pa_i3_3"
  p_adder2/3(out2) -> p_adder3/3(in2)  "pa_i3_2"
  p_adder2/2(out1) -> p_adder3/2(in1)  "pa_i3_1"
  p_adder1/5(out4) -> p_adder2/5(in4)  "pa_i2_4"
  p_adder1/4(out3) -> p_adder2/4(in3)  "pa_i2_3"
  p_adder1/3(out2) -> p_adder2/3(in2)  "pa_i2_2"
  p_adder1/2(out1) -> p_adder2/2(in1)  "pa_i2_1"
  in4/1 -> p_adder1/5(in4)
  in3/1 -> p_adder1/4(in3)
  in2/1 -> p_adder1/3(in2)
  in1/1 -> p_adder1/2(in1)

== system l8_ver130/s2/cic_pow2_2/Stage4_dec2 ==
  in1 : Inport
  in2 : Inport  Port=2
  sync_in : Inport  Port=3
  p_adder1 : Subsystem
      settings: AttributesFormatString=|
      | Accumulation Length = 2
      | Add Latency = 1
      | Delay Length =1
  p_adder2 : Subsystem
      settings: AttributesFormatString=|
      | Accumulation Length = 2
      | Add Latency = 1
      | Delay Length =1
  p_adder3 : Subsystem
      settings: AttributesFormatString=|
      | Accumulation Length = 2
      | Add Latency = 1
      | Delay Length =1
  p_adder4 : Subsystem
      settings: AttributesFormatString=|
      | Accumulation Length = 2
      | Add Latency = 1
      | Delay Length =1
  out1 : Outport
  sync_out : Outport  Port=2
  wires:
  sync_in/1 -> p_adder4/1(sync_in), p_adder3/1(sync_in), p_adder2/1(sync_in), p_adder1/1(sync_in)
  p_adder4/1(sync_out) -> sync_out/1  "sync_in5"
  p_adder4/3(out2) -> out1/1  "pa_i5_2"
  p_adder3/3(out2) -> p_adder4/3(in2)  "pa_i4_2"
  p_adder3/2(out1) -> p_adder4/2(in1)  "pa_i4_1"
  p_adder2/3(out2) -> p_adder3/3(in2)  "pa_i3_2"
  p_adder2/2(out1) -> p_adder3/2(in1)  "pa_i3_1"
  p_adder1/3(out2) -> p_adder2/3(in2)  "pa_i2_2"
  p_adder1/2(out1) -> p_adder2/2(in1)  "pa_i2_1"
  in2/1 -> p_adder1/3(in2)
  in1/1 -> p_adder1/2(in1)

== system l8_ver130/s2/cic_pow2_3/Stage1_dec2 ==
  in1 : Inport
  in2 : Inport  Port=2
  in3 : Inport  Port=3
  in4 : Inport  Port=4
  in5 : Inport  Port=5
  in6 : Inport  Port=6
  in7 : Inport  Port=7
  in8 : Inport  Port=8
  in9 : Inport  Port=9
  in10 : Inport  Port=10
  in11 : Inport  Port=11
  in12 : Inport  Port=12
  in13 : Inport  Port=13
  in14 : Inport  Port=14
  in15 : Inport  Port=15
  in16 : Inport  Port=16
  sync_in : Inport  Port=17
  p_adder1 : Subsystem
      settings: AttributesFormatString=|
      | Accumulation Length = 2
      | Add Latency = 1
      | Delay Length =1
  p_adder2 : Subsystem
      settings: AttributesFormatString=|
      | Accumulation Length = 2
      | Add Latency = 1
      | Delay Length =1
  p_adder3 : Subsystem
      settings: AttributesFormatString=|
      | Accumulation Length = 2
      | Add Latency = 1
      | Delay Length =1
  p_adder4 : Subsystem
      settings: AttributesFormatString=|
      | Accumulation Length = 2
      | Add Latency = 1
      | Delay Length =1
  out1 : Outport
  out2 : Outport  Port=2
  out3 : Outport  Port=3
  out4 : Outport  Port=4
  out5 : Outport  Port=5
  out6 : Outport  Port=6
  out7 : Outport  Port=7
  out8 : Outport  Port=8
  sync_out : Outport  Port=9
  wires:
  sync_in/1 -> p_adder4/1(sync_in), p_adder3/1(sync_in), p_adder2/1(sync_in), p_adder1/1(sync_in)
  p_adder4/1(sync_out) -> sync_out/1  "sync_in5"
  p_adder4/17(out16) -> out8/1  "pa_i5_16"
  p_adder4/15(out14) -> out7/1  "pa_i5_14"
  p_adder4/13(out12) -> out6/1  "pa_i5_12"
  p_adder4/11(out10) -> out5/1  "pa_i5_10"
  p_adder4/9(out8) -> out4/1  "pa_i5_8"
  p_adder4/7(out6) -> out3/1  "pa_i5_6"
  p_adder4/5(out4) -> out2/1  "pa_i5_4"
  p_adder4/3(out2) -> out1/1  "pa_i5_2"
  p_adder3/17(out16) -> p_adder4/17(in16)  "pa_i4_16"
  p_adder3/16(out15) -> p_adder4/16(in15)  "pa_i4_15"
  p_adder3/15(out14) -> p_adder4/15(in14)  "pa_i4_14"
  p_adder3/14(out13) -> p_adder4/14(in13)  "pa_i4_13"
  p_adder3/13(out12) -> p_adder4/13(in12)  "pa_i4_12"
  p_adder3/12(out11) -> p_adder4/12(in11)  "pa_i4_11"
  p_adder3/11(out10) -> p_adder4/11(in10)  "pa_i4_10"
  p_adder3/10(out9) -> p_adder4/10(in9)  "pa_i4_9"
  p_adder3/9(out8) -> p_adder4/9(in8)  "pa_i4_8"
  p_adder3/8(out7) -> p_adder4/8(in7)  "pa_i4_7"
  p_adder3/7(out6) -> p_adder4/7(in6)  "pa_i4_6"
  p_adder3/6(out5) -> p_adder4/6(in5)  "pa_i4_5"
  p_adder3/5(out4) -> p_adder4/5(in4)  "pa_i4_4"
  p_adder3/4(out3) -> p_adder4/4(in3)  "pa_i4_3"
  p_adder3/3(out2) -> p_adder4/3(in2)  "pa_i4_2"
  p_adder3/2(out1) -> p_adder4/2(in1)  "pa_i4_1"
  p_adder2/17(out16) -> p_adder3/17(in16)  "pa_i3_16"
  p_adder2/16(out15) -> p_adder3/16(in15)  "pa_i3_15"
  p_adder2/15(out14) -> p_adder3/15(in14)  "pa_i3_14"
  p_adder2/14(out13) -> p_adder3/14(in13)  "pa_i3_13"
  p_adder2/13(out12) -> p_adder3/13(in12)  "pa_i3_12"
  p_adder2/12(out11) -> p_adder3/12(in11)  "pa_i3_11"
  p_adder2/11(out10) -> p_adder3/11(in10)  "pa_i3_10"
  p_adder2/10(out9) -> p_adder3/10(in9)  "pa_i3_9"
  p_adder2/9(out8) -> p_adder3/9(in8)  "pa_i3_8"
  p_adder2/8(out7) -> p_adder3/8(in7)  "pa_i3_7"
  p_adder2/7(out6) -> p_adder3/7(in6)  "pa_i3_6"
  p_adder2/6(out5) -> p_adder3/6(in5)  "pa_i3_5"
  p_adder2/5(out4) -> p_adder3/5(in4)  "pa_i3_4"
  p_adder2/4(out3) -> p_adder3/4(in3)  "pa_i3_3"
  p_adder2/3(out2) -> p_adder3/3(in2)  "pa_i3_2"
  p_adder2/2(out1) -> p_adder3/2(in1)  "pa_i3_1"
  p_adder1/17(out16) -> p_adder2/17(in16)  "pa_i2_16"
  p_adder1/16(out15) -> p_adder2/16(in15)  "pa_i2_15"
  p_adder1/15(out14) -> p_adder2/15(in14)  "pa_i2_14"
  p_adder1/14(out13) -> p_adder2/14(in13)  "pa_i2_13"
  p_adder1/13(out12) -> p_adder2/13(in12)  "pa_i2_12"
  p_adder1/12(out11) -> p_adder2/12(in11)  "pa_i2_11"
  p_adder1/11(out10) -> p_adder2/11(in10)  "pa_i2_10"
  p_adder1/10(out9) -> p_adder2/10(in9)  "pa_i2_9"
  p_adder1/9(out8) -> p_adder2/9(in8)  "pa_i2_8"
  p_adder1/8(out7) -> p_adder2/8(in7)  "pa_i2_7"
  p_adder1/7(out6) -> p_adder2/7(in6)  "pa_i2_6"
  p_adder1/6(out5) -> p_adder2/6(in5)  "pa_i2_5"
  p_adder1/5(out4) -> p_adder2/5(in4)  "pa_i2_4"
  p_adder1/4(out3) -> p_adder2/4(in3)  "pa_i2_3"
  p_adder1/3(out2) -> p_adder2/3(in2)  "pa_i2_2"
  p_adder1/2(out1) -> p_adder2/2(in1)  "pa_i2_1"
  in16/1 -> p_adder1/17(in16)
  in15/1 -> p_adder1/16(in15)
  in14/1 -> p_adder1/15(in14)
  in13/1 -> p_adder1/14(in13)
  in12/1 -> p_adder1/13(in12)
  in11/1 -> p_adder1/12(in11)
  in10/1 -> p_adder1/11(in10)
  in9/1 -> p_adder1/10(in9)
  in8/1 -> p_adder1/9(in8)
  in7/1 -> p_adder1/8(in7)
  in6/1 -> p_adder1/7(in6)
  in5/1 -> p_adder1/6(in5)
  in4/1 -> p_adder1/5(in4)
  in3/1 -> p_adder1/4(in3)
  in2/1 -> p_adder1/3(in2)
  in1/1 -> p_adder1/2(in1)
